simulink model "px8d1k_1g"  (R2013a) (continued)
== system px8d1k_1g == (continued)
      mask prompts: n_streams "Number simultaneous streams"; FFTSize "Size of FFT: (2^? pts)"; n_inputs "Number of Simultaneous Inputs: (2^?)"; input_bit_width "Input Bit Width"; bin_pt_in "Input binary point"; coeff_bit_width "Coefficient Bit Width"; unscramble "Unscramble output (ie, put channels in canonical order)"; async "Asynchronous operation"; add_latency "Add Latency"; mult_latency "Mult Latency"; bram_latency "BRAM Latency"; conv_latency "Convert Latency"; input_latency "Input Latency"; biplex_direct_latency "Latency between biplexes and fft_direct"; quantization "Quantization Behavior"; overflow "Overflow Behavior"; delays_bit_limit "Number bits above which to store stage's delays in BRAM (2^? bits)"; coeffs_bit_limit "Number bits above which to store stage's coefficients in BRAM (2^? bits)"; coeff_sharing "BRAM sharing in coeff storage"; coeff_decimation "Store a fraction of coeff factors where useful"; coeff_generation "Generate coeffs with multipliers where useful"; cal_bits "Number calibration locations when generating coeffs (2^?)"; n_bits_rotation "Feedback rotation vector resolution"; mult_spec "Multiplier impl. (0=core, 1=embedded, 2=behavioural) (left=1st stage, or just one value)"; max_fanout "Maximum fanout"; bitgrowth "Bitgrowth instead of shifting"; max_bits "Max bits to grow to"; hardcode_shifts "Hardcode shift schedule"; shift_schedule "Shift schedule"; dsp48_adders "DSP48 adders in butterfly"
      mask help: A real-sampled wideband FFT.
      mask init:
      | fft_wideband_real_init(gcb, ...
      |     'n_streams', n_streams, ...
      |     'FFTSize', FFTSize, ...
      |     'n_inputs', n_inputs, ...
      |     'input_bit_width', input_bit_width, ...
      |     'bin_pt_in', bin_pt_in, ...
      |     'coeff_bit_width', coeff_bit_width, ...
      |     'unscramble', unscramble, ...
      |     'async', async, ...
      |     'add_latency', add_latency, ...
      |     'mult_latency', mult_latency, ...
      |     'bram_latency', bram_latency, ...
      |     'conv_latency', conv_latency, ...
      |     'input_latency', input_latency, ...
      |     'biplex_direct_latency', biplex_direct_latency, ...
      |     'quantization', quantization, ...
      |     'overflow', overflow, ...
      |     'delays_bit_limit', delays_bit_limit, ...
      |     'coeffs_bit_limit', coeffs_bit_limit, ...
      |     'coeff_sharing', coeff_sharing, ...
      |     'coeff_decimation', coeff_decimation, ...
      |     'coeff_generation', coeff_generation, ...
      |     'cal_bits', cal_bits, ...
      |     'n_bits_rotation', n_bits_rotation, ...
      |     'max_fanout', max_fanout, ...   
      |     'mult_spec', mult_spec, ...
      |     'bitgrowth', bitgrowth, ...
      |     'max_bits', max_bits, ...
      |     'hardcode_shifts', hardcode_shifts, ...
      |     'shift_schedule', shift_schedule, ...
      |     'dsp48_adders', dsp48_adders);
      settings: AttributesFormatString=|
      | 11 stages
      | (18,18)
      | Round  (unbiased: +/- Inf)
      | Saturate
      mask Help: eval('xlWeb(''http://casper.berkeley.edu/wiki/Fft_wideband_real'')')
      mask TypeOptions quantization: Truncate | Round  (unbiased: +/- Inf) | Round  (unbiased: Even Values)
      mask TypeOptions overflow: Wrap | Saturate | Error
      mask Callback hardcode_shifts:
      | hardcode_shifts = get_param(gcb, 'hardcode_shifts');
      | set_param_state(gcb, 'shift_schedule', hardcode_shifts);
      mask Callback dsp48_adders: fft_callback_dsp48_adders(gcb);
  gain0 : Subsystem  Tag=xps:sw_reg  AncestorBlock=xps_library/software register  mask(swreg){mode=one value, io_dir=From Processor, io_delay=0, sample_period=1, names=reg, bitwidths=32, arith_types=0, bin_pts=0, sim_port=on, show_format=off}
      mask prompts: io_dir "I/O direction"; io_delay "I/O delay"; sample_period "Sample period"; names "Name:"; bitwidths "Bitwidth:"; arith_types "Type (ufix=0, fix=1, bool=2):"; bin_pts "Binary pt:"; sim_port "Provide sim input/output?"; show_format "Print format string?"
      mask init:
      | swreg_init(gcb)
      settings: AttributesFormatString=1input
      mask TypeOptions mode: one value | fields of equal size | fields of arbitrary size
      mask Callback mode: swreg_cb(gcb);
      mask TypeOptions io_dir: To Processor | From Processor
      mask Callback io_dir:
      | mask_enables = get_param(gcb, 'MaskEnables');
      | mask_names = get_param(gcb, 'MaskNames');
      | if strcmp(get_param(gcb, 'io_dir'), 'To Processor'),
      |     mask_enables{ismember(mask_names, 'sample_period')} = 'off';
      | else
      |     mask_enables{ismember(mask_names, 'sample_period')} = 'on';
      | end
      | set_param(gcb, 'MaskEnables', mask_enables);
      mask Callback bitwidths:
      | swreg_maskcheck(gcb);
      | % blk = gcb;
      | % mode = get_param(blk, 'mode');
      | % current_widths = eval(get_param(blk, 'bitwidths'));
      | % if (strcmp(mode, 'one value') || strcmp(mode, 'fields of equal size')) && (length(current_widths) > 1),
      | %     error('Only one width may be specified for this mode.');
      | % elseif strcmp(mode, 'fields of arbitrary size')
      | %     current_names = textscan(strtrim(strrep(strrep(get_param(blk, 'names'), ']', ''), '[', '')), '%s');
      | %     current_names = current_names{1};
      | %     numios = length(current_names);
      | %     if length(current_widths) ~= numios,
      | %         error('%d field names specified, but %d widths?', numios, length(current_widths));
      | %     end
      | % end
      | % total_width = 0;
      | % for ctr = 1 : length(current_widths),
      | %     total_width = total_width + current_widths(ctr);
      | % end
      | % if total_width > 32,
      | %     error('Total width must be 32 or less, set to %d.', total_width);
      | % end
      mask Callback arith_types:
      | [numios, ~, ~, ~, current_types] = swreg_maskcheck(gcb);
      | % blk = gcb;
      | % mode = get_param(blk, 'mode');
      | % current_types = eval(get_param(blk, 'arith_types'));
      | % numios = length(current_types);
      | % if (strcmp(mode, 'one value') || strcmp(mode, 'fields of equal size')) && (length(current_types) > 1),
      | %     error('Only one type may be specified for this mode.');
      | % elseif strcmp(mode, 'fields of arbitrary size')
      | %     current_names = textscan(strtrim(strrep(strrep(get_param(blk, 'names'), ']', ''), '[', '')), '%s');
      | %     current_names = current_names{1};
      | %     numios = length(current_names);
      | %     if length(current_types) ~= numios,
      | %         error('%d field names specified, but %d types?', numios, length(current_types));
      | %     end
      | % end
      | problem = 0;
      | for ctr = 1 : numios,
      |     t = current_types(ctr);
      |     if (t < 0) || (t > 2),
      |         problem = t;
      |         ctr = numios + 1;
      |     end
      | end
      | if problem ~= 0,
      |     error('Type cannot be %i, must be 0, 1 or 2.', problem);
      | end
      mask Callback bin_pts:
      | swreg_maskcheck(gcb);
      | % blk = gcb;
      | % mode = get_param(blk, 'mode');
      | % current_bins = eval(get_param(blk, 'bin_pts'));
      | % if (strcmp(mode, 'one value') || strcmp(mode, 'fields of equal size')) && (length(current_bins) > 1),
      | %     error('Only one binary pt may be specified for this mode.');
      | % elseif strcmp(mode, 'fields of arbitrary size')
      | %     current_names = textscan(strtrim(strrep(strrep(get_param(blk, 'names'), ']', ''), '[', '')), '%s');
      | %     current_names = current_names{1};
      | %     numios = length(current_names);
      | %     if length(current_bins) ~= numios,
      | %         error('%d field names specified, but %d binary pts?', numios, length(current_bins));
      | %     end
      | % end
  gain1 : Subsystem  Tag=xps:sw_reg  AncestorBlock=xps_library/software register  mask(swreg){mode=one value, io_dir=From Processor, io_delay=0, sample_period=1, names=reg, bitwidths=32, arith_types=0, bin_pts=0, sim_port=on, show_format=off}
      mask prompts: io_dir "I/O direction"; io_delay "I/O delay"; sample_period "Sample period"; names "Name:"; bitwidths "Bitwidth:"; arith_types "Type (ufix=0, fix=1, bool=2):"; bin_pts "Binary pt:"; sim_port "Provide sim input/output?"; show_format "Print format string?"
      mask init:
      | swreg_init(gcb)
      settings: AttributesFormatString=1input
      mask TypeOptions mode: one value | fields of equal size | fields of arbitrary size
      mask Callback mode: swreg_cb(gcb);
      mask TypeOptions io_dir: To Processor | From Processor
      mask Callback io_dir:
      | mask_enables = get_param(gcb, 'MaskEnables');
      | mask_names = get_param(gcb, 'MaskNames');
      | if strcmp(get_param(gcb, 'io_dir'), 'To Processor'),
      |     mask_enables{ismember(mask_names, 'sample_period')} = 'off';
      | else
      |     mask_enables{ismember(mask_names, 'sample_period')} = 'on';
      | end
      | set_param(gcb, 'MaskEnables', mask_enables);
      mask Callback bitwidths:
      | swreg_maskcheck(gcb);
      | % blk = gcb;
      | % mode = get_param(blk, 'mode');
      | % current_widths = eval(get_param(blk, 'bitwidths'));
      | % if (strcmp(mode, 'one value') || strcmp(mode, 'fields of equal size')) && (length(current_widths) > 1),
      | %     error('Only one width may be specified for this mode.');
      | % elseif strcmp(mode, 'fields of arbitrary size')
      | %     current_names = textscan(strtrim(strrep(strrep(get_param(blk, 'names'), ']', ''), '[', '')), '%s');
      | %     current_names = current_names{1};
      | %     numios = length(current_names);
      | %     if length(current_widths) ~= numios,
      | %         error('%d field names specified, but %d widths?', numios, length(current_widths));
      | %     end
      | % end
      | % total_width = 0;
      | % for ctr = 1 : length(current_widths),
      | %     total_width = total_width + current_widths(ctr);
      | % end
      | % if total_width > 32,
      | %     error('Total width must be 32 or less, set to %d.', total_width);
      | % end
      mask Callback arith_types:
      | [numios, ~, ~, ~, current_types] = swreg_maskcheck(gcb);
      | % blk = gcb;
      | % mode = get_param(blk, 'mode');
      | % current_types = eval(get_param(blk, 'arith_types'));
      | % numios = length(current_types);
      | % if (strcmp(mode, 'one value') || strcmp(mode, 'fields of equal size')) && (length(current_types) > 1),
      | %     error('Only one type may be specified for this mode.');
      | % elseif strcmp(mode, 'fields of arbitrary size')
      | %     current_names = textscan(strtrim(strrep(strrep(get_param(blk, 'names'), ']', ''), '[', '')), '%s');
      | %     current_names = current_names{1};
      | %     numios = length(current_names);
      | %     if length(current_types) ~= numios,
      | %         error('%d field names specified, but %d types?', numios, length(current_types));
      | %     end
      | % end
      | problem = 0;
      | for ctr = 1 : numios,
      |     t = current_types(ctr);
      |     if (t < 0) || (t > 2),
      |         problem = t;
      |         ctr = numios + 1;
      |     end
      | end
      | if problem ~= 0,
      |     error('Type cannot be %i, must be 0, 1 or 2.', problem);
      | end
      mask Callback bin_pts:
      | swreg_maskcheck(gcb);
      | % blk = gcb;
      | % mode = get_param(blk, 'mode');
      | % current_bins = eval(get_param(blk, 'bin_pts'));
      | % if (strcmp(mode, 'one value') || strcmp(mode, 'fields of equal size')) && (length(current_bins) > 1),
      | %     error('Only one binary pt may be specified for this mode.');
      | % elseif strcmp(mode, 'fields of arbitrary size')
      | %     current_names = textscan(strtrim(strrep(strrep(get_param(blk, 'names'), ']', ''), '[', '')), '%s');
      | %     current_names = current_names{1};
      | %     numios = length(current_names);
      | %     if length(current_bins) ~= numios,
      | %         error('%d field names specified, but %d binary pts?', numios, length(current_bins));
      | %     end
      | % end
  one_GbE : xps_library/one_GbE  Tag=xps:onegbe  local_en=on  dis_cpu_rx=off  dis_cpu_tx=off  cpu_promiscuous=off  local_mac=hex2dec('123456789abc')  local_ip=hex2dec('c0a8290a')  local_port=4321  local_gateway=1
  pfb_fir_real : Subsystem  Tag=casper:pfb_fir_real  AncestorBlock=casper_library_pfbs/pfb_fir_real  mask(pfb_fir_real){PFBSize=11, TotalTaps=4, WindowType=hamming, n_inputs=3, MakeBiplex=on, BitWidthIn=8, BitWidthOut=18, CoeffBitWidth=18, CoeffDistMem=off, add_latency=3, mult_latency=4, bram_latency=4, fan_latency=2, conv_latency=2, quantization=Round  (unbiased: Even Values), fwidth=1, mult_spec=2, adder_folding=off, adder_imp=Behavioral, coeffs_share=on}
      mask prompts: PFBSize "Size of PFB: (2^? pnts)"; TotalTaps "Total Number of Taps:"; WindowType "Windowing Function:"; n_inputs "Number of Simultaneous Inputs: (2^?)"; MakeBiplex "Make Biplex?"; BitWidthIn "Input Bitwidth:"; BitWidthOut "Output Bitwidth:"; CoeffBitWidth "Coefficient Bitwidth:"; CoeffDistMem "Use Distributed Memory for Coeffs"; add_latency "Add Latency"; mult_latency "Mult Latency"; bram_latency "BRAM Latency"; fan_latency "Fanout latency"; conv_latency "Convert latency"; quantization "Quantization Behavior"; fwidth "Bin Width Scaling (normal=1)"; mult_spec "Multiplier specification (0=core, 1=embedded, 2=behavioural) (left=1st tap, or just one value)"; adder_folding "Fold adders into DSPs"; adder_imp "Adder implementation"; coeffs_share "Share coefficients between polarisations (use less BRAM)?"
      mask help:
      | Fold adders into DSPs: Causes adders to be absorbed into DSP blocks (supported in Virtex5)
      | Adder implementation: Cores using Fabric or DSP48 or behavioral HDL
      mask init:
      | pfb_fir_real_init(gcb, ...
      |     'PFBSize', PFBSize, ...
      |     'TotalTaps', TotalTaps, ...
      |     'WindowType', WindowType, ...
      |     'n_inputs', n_inputs, ...
      |     'MakeBiplex', MakeBiplex, ...
      |     'BitWidthIn', BitWidthIn, ...
      |     'BitWidthOut', BitWidthOut, ...
      |     'CoeffBitWidth', CoeffBitWidth, ...
      |     'CoeffDistMem', CoeffDistMem, ...
      |     'add_latency', add_latency, ...
      |     'mult_latency', mult_latency, ...
      |     'bram_latency', bram_latency, ...
      |     'fan_latency', fan_latency, ...
      |     'conv_latency', conv_latency, ...
      |     'quantization', quantization, ...
      |     'fwidth', fwidth, ...
      |     'mult_spec', mult_spec, ...
      |     'adder_folding', adder_folding, ...
      |     'adder_imp', adder_imp, ...
      |     'coeffs_share', coeffs_share);
      settings: AttributesFormatString=taps=4, add_latency=3
      mask Help: eval('xlWeb(''http://casper.berkeley.edu/wiki/Pfb_fir_real'')')
      mask Callback TotalTaps:
      | %num_taps = eval(get_param(gcb, 'TotalTaps'));
      | %old_vec = eval(get_param(gcb, 'mult_spec'));=-0l
      | 
      | %vec = 2.*ones(1,num_taps);
      | %old_size = length(old_vec);
      | 
      | %if(old_size <= num_taps),
      | %    new_size = old_size;
      | %else,
      | %    new_size = num_taps;
      | %end
      | 
      | %for n = 1:new_size, vec(n) = old_vec(n); end
      | %set_param(gcb, 'mult_spec', mat2str(vec));
      mask TypeOptions WindowType: bartlett | barthannwin | blackman | blackmanharris | bohamwin | chebwin | flattopwin | gausswin | hamming | hann | kaiser | nuttallwin | parzenwin | rectwin | tukeywin | triang | userwindow
      mask TypeOptions quantization: Truncate | Round  (unbiased: +/- Inf) | Round  (unbiased: Even Values)
      mask TypeOptions adder_imp: Behavioral | Fabric | DSP48
  pipeline5 : xbsIndex_r4/Delay  rst=off  infoeditControl=Selection of Reset will increase slice count due to use of real FFs and instead of SRLs  en=off  latency=1  dbl_ovrd=off  reg_retiming=on  block_type=delay
      settings: xl_area=[0, 0, 0, 0, 0, 0, 0]  block_version=11.4
  pkt_64_to_8 : Subsystem  mask{pktlen=2^8, idlecycles=64}
      mask prompts: pktlen "Packet length in INPUT word (64bit):"; idlecycles "Idle cycles between packet:"
  power : Subsystem  mask{bitwidth=18, add_latency=2, mult_latency=3, mode=dsp48e, n_inputs=8}
      mask prompts: bitwidth "Bit Width"; add_latency "Adder Latency"; mult_latency "Mult Latency"; n_inputs "Number of Inputs"
      mask init:
      | config.source = str2func('spect_power');
      | config.depend = {'spect_power.m'};
      | config.toplevel = gcb;
      | xBlock(config, {bitwidth, add_latency, mult_latency, mode, n_inputs});
      mask TypeOptions mode: behavioral | dsp48e
  ppsarm : Subsystem
  prescale : Subsystem  mask{mult_latency=3}
      mask prompts: mult_latency "Mult Latency"
  reset : Subsystem  Tag=xps:sw_reg  AncestorBlock=xps_library/software register  mask(swreg){mode=one value, io_dir=From Processor, io_delay=0, sample_period=1, names=reg, bitwidths=32, arith_types=2, bin_pts=0, sim_port=on, show_format=off}
      mask prompts: io_dir "I/O direction"; io_delay "I/O delay"; sample_period "Sample period"; names "Name:"; bitwidths "Bitwidth:"; arith_types "Type (ufix=0, fix=1, bool=2):"; bin_pts "Binary pt:"; sim_port "Provide sim input/output?"; show_format "Print format string?"
      mask init:
      | swreg_init(gcb)
      settings: AttributesFormatString=1input
      mask TypeOptions mode: one value | fields of equal size | fields of arbitrary size
      mask Callback mode: swreg_cb(gcb);
      mask TypeOptions io_dir: To Processor | From Processor
      mask Callback io_dir:
      | mask_enables = get_param(gcb, 'MaskEnables');
      | mask_names = get_param(gcb, 'MaskNames');
      | if strcmp(get_param(gcb, 'io_dir'), 'To Processor'),
      |     mask_enables{ismember(mask_names, 'sample_period')} = 'off';
      | else
      |     mask_enables{ismember(mask_names, 'sample_period')} = 'on';
      | end
      | set_param(gcb, 'MaskEnables', mask_enables);
      mask Callback bitwidths:
      | swreg_maskcheck(gcb);
      | % blk = gcb;
      | % mode = get_param(blk, 'mode');
      | % current_widths = eval(get_param(blk, 'bitwidths'));
      | % if (strcmp(mode, 'one value') || strcmp(mode, 'fields of equal size')) && (length(current_widths) > 1),
      | %     error('Only one width may be specified for this mode.');
      | % elseif strcmp(mode, 'fields of arbitrary size')
      | %     current_names = textscan(strtrim(strrep(strrep(get_param(blk, 'names'), ']', ''), '[', '')), '%s');
      | %     current_names = current_names{1};
      | %     numios = length(current_names);
      | %     if length(current_widths) ~= numios,
      | %         error('%d field names specified, but %d widths?', numios, length(current_widths));
      | %     end
      | % end
      | % total_width = 0;
      | % for ctr = 1 : length(current_widths),
      | %     total_width = total_width + current_widths(ctr);
      | % end
      | % if total_width > 32,
      | %     error('Total width must be 32 or less, set to %d.', total_width);
      | % end
      mask Callback arith_types:
      | [numios, ~, ~, ~, current_types] = swreg_maskcheck(gcb);
      | % blk = gcb;
      | % mode = get_param(blk, 'mode');
      | % current_types = eval(get_param(blk, 'arith_types'));
      | % numios = length(current_types);
      | % if (strcmp(mode, 'one value') || strcmp(mode, 'fields of equal size')) && (length(current_types) > 1),
      | %     error('Only one type may be specified for this mode.');
      | % elseif strcmp(mode, 'fields of arbitrary size')
      | %     current_names = textscan(strtrim(strrep(strrep(get_param(blk, 'names'), ']', ''), '[', '')), '%s');
      | %     current_names = current_names{1};
      | %     numios = length(current_names);
      | %     if length(current_types) ~= numios,
      | %         error('%d field names specified, but %d types?', numios, length(current_types));
      | %     end
      | % end
      | problem = 0;
      | for ctr = 1 : numios,
      |     t = current_types(ctr);
      |     if (t < 0) || (t > 2),
      |         problem = t;
      |         ctr = numios + 1;
      |     end
      | end
      | if problem ~= 0,
      |     error('Type cannot be %i, must be 0, 1 or 2.', problem);
      | end
      mask Callback bin_pts:
      | swreg_maskcheck(gcb);
      | % blk = gcb;
      | % mode = get_param(blk, 'mode');
      | % current_bins = eval(get_param(blk, 'bin_pts'));
      | % if (strcmp(mode, 'one value') || strcmp(mode, 'fields of equal size')) && (length(current_bins) > 1),
      | %     error('Only one binary pt may be specified for this mode.');
      | % elseif strcmp(mode, 'fields of arbitrary size')
      | %     current_names = textscan(strtrim(strrep(strrep(get_param(blk, 'names'), ']', ''), '[', '')), '%s');
      | %     current_names = current_names{1};
      | %     numios = length(current_names);
      | %     if length(current_bins) ~= numios,
      | %         error('%d field names specified, but %d binary pts?', numios, length(current_bins));
      | %     end
      | % end
  scope0 : Subsystem  Tag=casper:snapshot  AncestorBlock=casper_library_scopes/snapshot  mask(snapshot){storage=bram, dram_dimm=1, dram_clock=200, nsamples=8, data_width=128, offset=off, circap=off, value=off, use_dsp48=on}
      mask prompts: storage "Storage medium"; dram_dimm "DRAM dimm"; dram_clock "DRAM clock rate"; nsamples "Number of Samples (2^?)"; data_width "Data width"; offset "Start delay support"; circap "Circular capture support"; value "Extra value capture support"; use_dsp48 "Use DSP48s to implement counters"
      mask help:
      | Standard snap block, but with the following optional extras;
      |  1) Optionally delay the fabric trigger by a user-specified number of valids.
      |  2) Capture continuously after a trigger until a stop pulse is received. Data is captured into BRAM continuously, with old data being overwritten until a stop command is received. Then stop capturing when memory is filled. This enables you to get some data before and some data after the stop pulse. Use the 'tr_en_cnt' register to determine where you are in your vector. 'Addr' register's MSb now indicates 'busy capturing'.
      |  3) Optionally capture a value on an input port to a register at the same time as the first data sample.
      |  4) Implement counters using DSP48Es to save resources.
      mask init: same as px8d1k_1g/adc_scope0
      mask TypeOptions storage: bram | dram
      mask Callback storage: snapshot_callback();
      mask TypeOptions dram_dimm: 1 | 2 | 3 | 4
      mask TypeOptions dram_clock: 200 | 250 | 266 | 300 | 333
      mask TypeOptions data_width: 8 | 16 | 32 | 64 | 128
  scope1 : Subsystem  Tag=casper:snapshot  AncestorBlock=casper_library_scopes/snapshot  mask(snapshot){storage=bram, dram_dimm=1, dram_clock=200, nsamples=8, data_width=128, offset=off, circap=off, value=off, use_dsp48=on}
      mask prompts: storage "Storage medium"; dram_dimm "DRAM dimm"; dram_clock "DRAM clock rate"; nsamples "Number of Samples (2^?)"; data_width "Data width"; offset "Start delay support"; circap "Circular capture support"; value "Extra value capture support"; use_dsp48 "Use DSP48s to implement counters"
      mask help:
      | Standard snap block, but with the following optional extras;
      |  1) Optionally delay the fabric trigger by a user-specified number of valids.
      |  2) Capture continuously after a trigger until a stop pulse is received. Data is captured into BRAM continuously, with old data being overwritten until a stop command is received. Then stop capturing when memory is filled. This enables you to get some data before and some data after the stop pulse. Use the 'tr_en_cnt' register to determine where you are in your vector. 'Addr' register's MSb now indicates 'busy capturing'.
      |  3) Optionally capture a value on an input port to a register at the same time as the first data sample.
      |  4) Implement counters using DSP48Es to save resources.
      mask init: same as px8d1k_1g/adc_scope0
      mask TypeOptions storage: bram | dram
      mask Callback storage: snapshot_callback();
      mask TypeOptions dram_dimm: 1 | 2 | 3 | 4
      mask TypeOptions dram_clock: 200 | 250 | 266 | 300 | 333
      mask TypeOptions data_width: 8 | 16 | 32 | 64 | 128
  sim_acclen : Constant  Value=2
  sim_bitsel : Constant
  sim_destip : Constant  Value=10*2^24+123
  sim_destport : Constant  Value=33333
  sim_fftshift : Constant  Value=2^32-1
  sim_gain0 : Constant  Value=2^12
  sim_gain1 : Constant  Value=2^12
  sim_usetvg : Constant
  status : Subsystem  Tag=xps:sw_reg  AncestorBlock=xps_library/software register  mask(swreg){mode=fields of arbitrary size, io_dir=To Processor, io_delay=0, sample_period=1, names=[unused tx_overrun pkt_overrun fft_overflow], bitwidths=[26 1 1 4], arith_types=[0 2 2 0], bin_pts=0, sim_port=off, show_format=off}
      mask prompts: io_dir "I/O direction"; io_delay "I/O delay"; sample_period "Sample period"; names "Names [msb ... lsb]:"; bitwidths "Bitwidths [msb ... lsb]:"; arith_types "Types (ufix=0, fix=1, bool=2) [msb ... lsb]:"; bin_pts "Binary pts [msb ... lsb]:"; sim_port "Provide sim input/output?"; show_format "Print format string?"
      mask init:
      | swreg_init(gcb)
      settings: AttributesFormatString=4 outputs
      mask TypeOptions mode: one value | fields of equal size | fields of arbitrary size
      mask Callback mode: swreg_cb(gcb);
      mask TypeOptions io_dir: To Processor | From Processor
      mask Callback io_dir:
      | mask_enables = get_param(gcb, 'MaskEnables');
      | mask_names = get_param(gcb, 'MaskNames');
      | if strcmp(get_param(gcb, 'io_dir'), 'To Processor'),
      |     mask_enables{ismember(mask_names, 'sample_period')} = 'off';
      | else
      |     mask_enables{ismember(mask_names, 'sample_period')} = 'on';
      | end
      | set_param(gcb, 'MaskEnables', mask_enables);
      mask Callback bitwidths:
      | swreg_maskcheck(gcb);
      | % blk = gcb;
      | % mode = get_param(blk, 'mode');
      | % current_widths = eval(get_param(blk, 'bitwidths'));
      | % if (strcmp(mode, 'one value') || strcmp(mode, 'fields of equal size')) && (length(current_widths) > 1),
      | %     error('Only one width may be specified for this mode.');
      | % elseif strcmp(mode, 'fields of arbitrary size')
      | %     current_names = textscan(strtrim(strrep(strrep(get_param(blk, 'names'), ']', ''), '[', '')), '%s');
      | %     current_names = current_names{1};
      | %     numios = length(current_names);
      | %     if length(current_widths) ~= numios,
      | %         error('%d field names specified, but %d widths?', numios, length(current_widths));
      | %     end
      | % end
      | % total_width = 0;
      | % for ctr = 1 : length(current_widths),
      | %     total_width = total_width + current_widths(ctr);
      | % end
      | % if total_width > 32,
      | %     error('Total width must be 32 or less, set to %d.', total_width);
      | % end
      mask Callback arith_types:
      | [numios, ~, ~, ~, current_types] = swreg_maskcheck(gcb);
      | % blk = gcb;
      | % mode = get_param(blk, 'mode');
      | % current_types = eval(get_param(blk, 'arith_types'));
      | % numios = length(current_types);
      | % if (strcmp(mode, 'one value') || strcmp(mode, 'fields of equal size')) && (length(current_types) > 1),
      | %     error('Only one type may be specified for this mode.');
      | % elseif strcmp(mode, 'fields of arbitrary size')
      | %     current_names = textscan(strtrim(strrep(strrep(get_param(blk, 'names'), ']', ''), '[', '')), '%s');
      | %     current_names = current_names{1};
      | %     numios = length(current_names);
      | %     if length(current_types) ~= numios,
      | %         error('%d field names specified, but %d types?', numios, length(current_types));
      | %     end
      | % end
      | problem = 0;
      | for ctr = 1 : numios,
      |     t = current_types(ctr);
      |     if (t < 0) || (t > 2),
      |         problem = t;
      |         ctr = numios + 1;
      |     end
      | end
      | if problem ~= 0,
      |     error('Type cannot be %i, must be 0, 1 or 2.', problem);
      | end
      mask Callback bin_pts:
      | swreg_maskcheck(gcb);
      | % blk = gcb;
      | % mode = get_param(blk, 'mode');
      | % current_bins = eval(get_param(blk, 'bin_pts'));
      | % if (strcmp(mode, 'one value') || strcmp(mode, 'fields of equal size')) && (length(current_bins) > 1),
      | %     error('Only one binary pt may be specified for this mode.');
      | % elseif strcmp(mode, 'fields of arbitrary size')
      | %     current_names = textscan(strtrim(strrep(strrep(get_param(blk, 'names'), ']', ''), '[', '')), '%s');
      | %     current_names = current_names{1};
      | %     numios = length(current_names);
      | %     if length(current_bins) ~= numios,
      | %         error('%d field names specified, but %d binary pts?', numios, length(current_bins));
      | %     end
      | % end
  step_arm : Step  After=7  SampleTime=0
  step_reset : Step  SampleTime=0
  use_tvg : Subsystem  Tag=xps:sw_reg  AncestorBlock=xps_library/software register  mask(swreg){mode=one value, io_dir=From Processor, io_delay=0, sample_period=1, names=reg, bitwidths=32, arith_types=2, bin_pts=0, sim_port=on, show_format=off}
      mask prompts: io_dir "I/O direction"; io_delay "I/O delay"; sample_period "Sample period"; names "Name:"; bitwidths "Bitwidth:"; arith_types "Type (ufix=0, fix=1, bool=2):"; bin_pts "Binary pt:"; sim_port "Provide sim input/output?"; show_format "Print format string?"
      mask init:
      | swreg_init(gcb)
      settings: AttributesFormatString=1input
      mask TypeOptions mode: one value | fields of equal size | fields of arbitrary size
      mask Callback mode: swreg_cb(gcb);
      mask TypeOptions io_dir: To Processor | From Processor
      mask Callback io_dir:
      | mask_enables = get_param(gcb, 'MaskEnables');
      | mask_names = get_param(gcb, 'MaskNames');
      | if strcmp(get_param(gcb, 'io_dir'), 'To Processor'),
      |     mask_enables{ismember(mask_names, 'sample_period')} = 'off';
      | else
      |     mask_enables{ismember(mask_names, 'sample_period')} = 'on';
      | end
      | set_param(gcb, 'MaskEnables', mask_enables);
      mask Callback bitwidths:
      | swreg_maskcheck(gcb);
      | % blk = gcb;
      | % mode = get_param(blk, 'mode');
      | % current_widths = eval(get_param(blk, 'bitwidths'));
      | % if (strcmp(mode, 'one value') || strcmp(mode, 'fields of equal size')) && (length(current_widths) > 1),
      | %     error('Only one width may be specified for this mode.');
      | % elseif strcmp(mode, 'fields of arbitrary size')
      | %     current_names = textscan(strtrim(strrep(strrep(get_param(blk, 'names'), ']', ''), '[', '')), '%s');
      | %     current_names = current_names{1};
      | %     numios = length(current_names);
      | %     if length(current_widths) ~= numios,
      | %         error('%d field names specified, but %d widths?', numios, length(current_widths));
      | %     end
      | % end
      | % total_width = 0;
      | % for ctr = 1 : length(current_widths),
      | %     total_width = total_width + current_widths(ctr);
      | % end
      | % if total_width > 32,
      | %     error('Total width must be 32 or less, set to %d.', total_width);
      | % end
      mask Callback arith_types:
      | [numios, ~, ~, ~, current_types] = swreg_maskcheck(gcb);
      | % blk = gcb;
      | % mode = get_param(blk, 'mode');
      | % current_types = eval(get_param(blk, 'arith_types'));
      | % numios = length(current_types);
      | % if (strcmp(mode, 'one value') || strcmp(mode, 'fields of equal size')) && (length(current_types) > 1),
      | %     error('Only one type may be specified for this mode.');
      | % elseif strcmp(mode, 'fields of arbitrary size')
      | %     current_names = textscan(strtrim(strrep(strrep(get_param(blk, 'names'), ']', ''), '[', '')), '%s');
      | %     current_names = current_names{1};
      | %     numios = length(current_names);
      | %     if length(current_types) ~= numios,
      | %         error('%d field names specified, but %d types?', numios, length(current_types));
      | %     end
      | % end
      | problem = 0;
      | for ctr = 1 : numios,
      |     t = current_types(ctr);
      |     if (t < 0) || (t > 2),
      |         problem = t;
      |         ctr = numios + 1;
      |     end
      | end
      | if problem ~= 0,
      |     error('Type cannot be %i, must be 0, 1 or 2.', problem);
      | end
      mask Callback bin_pts:
      | swreg_maskcheck(gcb);
      | % blk = gcb;
      | % mode = get_param(blk, 'mode');
      | % current_bins = eval(get_param(blk, 'bin_pts'));
      | % if (strcmp(mode, 'one value') || strcmp(mode, 'fields of equal size')) && (length(current_bins) > 1),
      | %     error('Only one binary pt may be specified for this mode.');
      | % elseif strcmp(mode, 'fields of arbitrary size')
      | %     current_names = textscan(strtrim(strrep(strrep(get_param(blk, 'names'), ']', ''), '[', '')), '%s');
      | %     current_names = current_names{1};
      | %     numios = length(current_names);
      | %     if length(current_bins) ~= numios,
      | %         error('%d field names specified, but %d binary pts?', numios, length(current_bins));
      | %     end
      | % end
  vacc : Subsystem  AncestorBlock=gavrt_library/vacc  mask(vacc){veclen=8, ninputs=8, max_accum=20, arith_type=0, in_bit_width=36, in_bin_pt=34, out_bit_width=32, out_bin_pt=23, register_din=1, add_latency=3, bram_latency=3, mux_latency=2}
      mask prompts: veclen "Vector Length (2^?)"; ninputs "Number of Simultaneous Vectors"; max_accum "Maximum # Accumulations (2^?)"; arith_type "Arithmetic Type (0=Unsigned, 1=Signed)"; in_bit_width "Input Bit Width"; in_bin_pt "Input Binary Point"; out_bit_width "Output Bit Width"; out_bin_pt "Output Binary Point"; register_din "Register Input Data  (1 = Enable Register Retiming on input delay)"; add_latency "Add Latency"; bram_latency "BRAM Latency"; mux_latency "Mux Latency"
      mask help:
      | Sync is expected the clock before the first data to be accumulated.
      | Use the Cram block to feed din for multiple simultaneous inputs.
      | acc_len is the number of vectors to sum.
      | valid is high while a valid accumulation is being clocked out on dout.
      | If only one sync pulse is provided, valid accumulations will repeatedly appear on dout as they become available.
      | Undesired behavior may occur if acc_len is changed without a new sync pulse.
      | Note: The output is an unsigned concatenation of the output streams. Thus, even with only one stream, the output must be recast before using.
      | Register Input Data controls the "Enable Register Retiming" parameter of the delays on 'din'.
      mask init:
      | vacc_init(gcb, ...
      |     'veclen', veclen, ...
      |     'ninputs', ninputs, ...
      |     'max_accum', max_accum, ...
      |     'arith_type', arith_type, ...
      |     'in_bit_width', in_bit_width, ...
      |     'in_bin_pt', in_bin_pt, ...
      |     'out_bit_width', out_bit_width, ...
      |     'out_bin_pt', out_bin_pt, ...
      |     'register_din', register_din, ...
      |     'add_latency', add_latency, ...
      |     'bram_latency', bram_latency, ...
      |     'mux_latency', mux_latency);
      settings: AttributesFormatString=|
      | vector length=256, inputs=8
      | max accumulations=2^20
  wires:
  step_reset/1 -> reset/1(sim_1)
  reset/1(in_reg) -> Delay19/1
  edge_detect/1 -> [sys_reset]
  [adc_in] -> bus_expand/1(bus_in)
  Sine Wave1/1 -> asiaa_adc5g/1(sim_a)
  Pulse Generator1/1 -> asiaa_adc5g/3(sim_sync)
  asiaa_adc5g/1(a0) -> Delay2/1
  asiaa_adc5g/2(a1) -> Delay3/1
  asiaa_adc5g/3(a2) -> Delay4/1
  asiaa_adc5g/4(a3) -> Delay5/1
  asiaa_adc5g/5(a4) -> Delay6/1
  asiaa_adc5g/6(a5) -> Delay7/1
  asiaa_adc5g/7(a6) -> Delay8/1
  asiaa_adc5g/8(a7) -> Delay9/1
  asiaa_adc5g/14(c5) -> Delay15/1
  asiaa_adc5g/16(c7) -> Delay17/1
  asiaa_adc5g/13(c4) -> Delay14/1
  asiaa_adc5g/12(c3) -> Delay13/1
  asiaa_adc5g/15(c6) -> Delay16/1
  asiaa_adc5g/11(c2) -> Delay12/1
  asiaa_adc5g/10(c1) -> Delay11/1
  asiaa_adc5g/9(c0) -> Delay10/1
  conv_obbus/11(q10) -> bus_create1/11(in11)
  conv_obbus/9(q8) -> bus_create1/9(in9)
  conv_obbus/10(q9) -> bus_create1/10(in10)
  conv_obbus/14(q13) -> bus_create1/14(in14)
  conv_obbus/15(q14) -> bus_create1/15(in15)
  conv_obbus/16(q15) -> bus_create1/16(in16)
  conv_obbus/13(q12) -> bus_create1/13(in13)
  conv_obbus/12(q11) -> bus_create1/12(in12)
  conv_obbus/1(q0) -> bus_create1/1(in1)
  conv_obbus/2(q1) -> bus_create1/2(in2)
  conv_obbus/3(q2) -> bus_create1/3(in3)
  conv_obbus/4(q3) -> bus_create1/4(in4)
  conv_obbus/5(q4) -> bus_create1/5(in5)
  conv_obbus/6(q5) -> bus_create1/6(in6)
  conv_obbus/7(q6) -> bus_create1/7(in7)
  conv_obbus/8(q7) -> bus_create1/8(in8)
  bus_create1/1(bus_out) -> Delay29/1
  [reset] -> pfb_fir_real/1(sync)
  bus_expand/2(out15) -> pfb_fir_real/3(pol1_in2)
  bus_expand/1(msb_out16) -> pfb_fir_real/2(pol1_in1)
  bus_expand/10(out7) -> pfb_fir_real/11(pol2_in2)
  bus_expand/11(out6) -> pfb_fir_real/12(pol2_in3)
  bus_expand/12(out5) -> pfb_fir_real/13(pol2_in4)
  bus_expand/13(out4) -> pfb_fir_real/14(pol2_in5)
  bus_expand/14(out3) -> pfb_fir_real/15(pol2_in6)
  bus_expand/15(out2) -> pfb_fir_real/16(pol2_in7)
  bus_expand/16(lsb_out1) -> pfb_fir_real/17(pol2_in8)
  bus_expand/9(out8) -> pfb_fir_real/10(pol2_in1)
  bus_expand/4(out13) -> pfb_fir_real/5(pol1_in4)
  bus_expand/8(out9) -> pfb_fir_real/9(pol1_in8)
  bus_expand/3(out14) -> pfb_fir_real/4(pol1_in3)
  bus_expand/6(out11) -> pfb_fir_real/7(pol1_in6)
  bus_expand/5(out12) -> pfb_fir_real/6(pol1_in5)
  bus_expand/7(out10) -> pfb_fir_real/8(pol1_in7)
  pfb_fir_real/1(sync_out) -> fft_wideband_real/1(sync)
  [fft_shift] -> fft_wideband_real/2(shift)
  pfb_fir_real/17(pol2_out8) -> fft_wideband_real/18(in17)
  pfb_fir_real/16(pol2_out7) -> fft_wideband_real/17(in16)
  pfb_fir_real/15(pol2_out6) -> fft_wideband_real/16(in15)
  pfb_fir_real/14(pol2_out5) -> fft_wideband_real/15(in14)
  pfb_fir_real/13(pol2_out4) -> fft_wideband_real/14(in13)
  pfb_fir_real/12(pol2_out3) -> fft_wideband_real/13(in12)
  pfb_fir_real/11(pol2_out2) -> fft_wideband_real/12(in11)
  pfb_fir_real/10(pol2_out1) -> fft_wideband_real/11(in10)
  pfb_fir_real/2(pol1_out1) -> fft_wideband_real/3(in00)
  pfb_fir_real/3(pol1_out2) -> fft_wideband_real/4(in01)
  pfb_fir_real/4(pol1_out3) -> fft_wideband_real/5(in02)
  pfb_fir_real/5(pol1_out4) -> fft_wideband_real/6(in03)
  pfb_fir_real/6(pol1_out5) -> fft_wideband_real/7(in04)
  pfb_fir_real/8(pol1_out7) -> fft_wideband_real/9(in06)
  pfb_fir_real/7(pol1_out6) -> fft_wideband_real/8(in05)
  pfb_fir_real/9(pol1_out8) -> fft_wideband_real/10(in07)
  fft_wideband_real/1(sync_out) -> prescale/3(sync_in)
  fft_wideband_real/2(out00) -> prescale/4(din00)
  fft_wideband_real/3(out01) -> prescale/5(din01)
  fft_wideband_real/4(out02) -> prescale/6(din02)
  fft_wideband_real/5(out03) -> prescale/7(din03)
  fft_wideband_real/6(out10) -> prescale/8(din10)
  fft_wideband_real/7(out11) -> prescale/9(din11)
  fft_wideband_real/8(out12) -> prescale/10(din12)
  fft_wideband_real/9(out13) -> prescale/11(din13)
  prescale/5(dout03) -> power/5(din_3)
  prescale/4(dout02) -> power/4(din_2)
  prescale/6(dout10) -> power/6(din_4)
  prescale/8(dout12) -> power/8(din_6)
  prescale/9(dout13) -> power/9(din_7)
  prescale/7(dout11) -> power/7(din_5)
  prescale/1(sync_out) -> power/1(sync_in)
  prescale/3(dout01) -> power/3(din_1)
  prescale/2(dout00) -> power/2(din_0)
  gain0/1(in_reg) -> Delay23/1
  gain1/1(in_reg) -> Delay24/1
  sim_gain0/1 -> gain0/1(sim_1)
  sim_gain1/1 -> gain1/1(sim_1)
  [gain0] -> prescale/1(coeff0)
  [gain1] -> prescale/2(coeff1)
  power/1(sync_out) -> Gateway Out29/1, vacc/1(sync), Delay30/1
  bus_create2/1(bus_out) -> bus_expand3/1(bus_in), vacc/2(din)
  power/2(dout_0) -> bus_create2/1(in1)
  power/3(dout_1) -> bus_create2/2(in2)
  power/4(dout_2) -> bus_create2/3(in3)
  power/5(dout_3) -> bus_create2/4(in4)
  power/6(dout_4) -> bus_create2/5(in5)
  power/7(dout_5) -> bus_create2/6(in6)
  power/8(dout_6) -> bus_create2/7(in7)
  power/9(dout_7) -> bus_create2/8(in8)
  acc_len/1(in_reg) -> Delay25/1
  sim_acclen/1 -> acc_len/1(sim_1)
  [acc_len] -> vacc/3(acc_len)
  vacc/1(valid) -> [vacc_valid]
  vacc/2(dout) -> bitselect/2(data_in), Slice1/1, Slice6/1
  [vacc_valid] -> Delay/1, edge_detect1/1(in)
  edge_detect1/1(out) -> Gateway Out10/1, scope0/3(trig)
  Slice6/1 -> Delay32/1
  Delay/1 -> Gateway Out9/1, scope0/2(we)
  [vacc_valid] -> Delay1/1, edge_detect2/1(in)
  Slice1/1 -> Delay33/1
  Delay1/1 -> scope1/2(we)
  edge_detect2/1(out) -> scope1/3(trig)
  bit_select/1(in_reg) -> Delay22/1
  sim_bitsel/1 -> bit_select/1(sim_1)
  [bitsel] -> bitselect/1(bitselect)
  [vacc_valid] -> pipeline5/1
  pipeline5/1 -> pkt_64_to_8/3(din_valid)
  pkt_64_to_8/1(dout) -> Gateway Out3/1, one_GbE/3
  pkt_64_to_8/2(dout_valid) -> Gateway Out1/1, one_GbE/4
  pkt_64_to_8/3(eof) -> Gateway Out2/1, one_GbE/7
  pkt_64_to_8/4(overflow) -> [pkt_overrun]
  bitselect/1(data_out) -> pkt_64_to_8/2(din)
  [reset1] -> pkt_64_to_8/1(reset)
  dest_ip/1(in_reg) -> Delay18/1
  sim_destip/1 -> dest_ip/1(sim_1)
  dest_port/1(in_reg) -> Delay20/1
  sim_destport/1 -> dest_port/1(sim_1)
  fft_wideband_real/10(of) -> [fft_overflow]
  [fft_overflow] -> Delay26/1
  [pkt_overrun] -> Delay27/1
  [gbe_overrun] -> Delay28/1
  Constant2/1 -> status/1(out_unused)
  one_GbE/2 -> [gbe_overrun]
  [dest_ip] -> one_GbE/5
  [dest_port] -> one_GbE/6
  Constant3/1 -> one_GbE/2
  Constant4/1 -> one_GbE/8
  one_GbE/1 -> Terminator8/1
  one_GbE/3 -> Terminator1/1
  one_GbE/4 -> Terminator2/1
  one_GbE/5 -> Terminator3/1
  one_GbE/6 -> Terminator4/1
  one_GbE/7 -> Terminator5/1
  one_GbE/8 -> Terminator6/1
  one_GbE/9 -> Terminator7/1
  one_GbE/10 -> Terminator9/1
  one_GbE/11 -> Terminator10/1
  [adc5g] -> Slice2/1
  Constant5/1 -> adc_scope0/2(we), adc_scope0/3(trig)
  Slice2/1 -> adc_scope0/1(din)
  [adc5g] -> Slice3/1
  Constant6/1 -> adc_scope1/3(trig), adc_scope1/2(we)
  Slice3/1 -> adc_scope1/1(din)
  bus_expand2/11(out6) -> Gateway Out23/1
  bus_expand2/12(out5) -> Gateway Out24/1
  bus_expand2/2(out15) -> Gateway Out14/1
  bus_expand2/3(out14) -> Gateway Out15/1
  bus_expand2/5(out12) -> Gateway Out17/1
  bus_expand2/4(out13) -> Gateway Out16/1
  bus_expand2/8(out9) -> Gateway Out20/1
  bus_expand2/15(out2) -> Gateway Out27/1
  bus_expand2/14(out3) -> Gateway Out26/1
  bus_expand2/16(lsb_out1) -> Gateway Out28/1
  bus_expand2/10(out7) -> Gateway Out22/1
  bus_expand2/7(out10) -> Gateway Out19/1
  bus_expand2/9(out8) -> Gateway Out21/1
  bus_expand2/1(msb_out16) -> Gateway Out13/1
  bus_expand2/6(out11) -> Gateway Out18/1
  bus_expand2/13(out4) -> Gateway Out25/1
  [adc_in] -> bus_expand2/1(bus_in)
  bus_expand3/2(out7) -> Gateway Out31/1
  bus_expand3/5(out4) -> Gateway Out34/1
  bus_expand3/3(out6) -> Gateway Out32/1
  bus_expand3/4(out5) -> Gateway Out33/1
  bus_expand3/8(lsb_out1) -> Gateway Out37/1
  bus_expand3/1(msb_out8) -> Gateway Out30/1
  bus_expand3/7(out2) -> Gateway Out36/1
  bus_expand3/6(out3) -> Gateway Out35/1
  Gateway Out29/1 -> ScopeFFT/1
  Gateway Out30/1 -> ScopeFFT/2
  Gateway Out31/1 -> ScopeFFT/3
  Gateway Out32/1 -> ScopeFFT/4
  Gateway Out33/1 -> ScopeFFT/5
  Gateway Out34/1 -> ScopeFFT/6
  Gateway Out35/1 -> ScopeFFT/7
  Gateway Out36/1 -> ScopeFFT/8
  Gateway Out37/1 -> ScopeFFT/9
  Gateway Out1/1 -> ScopeGBE/1
  Gateway Out2/1 -> ScopeGBE/2
  Gateway Out3/1 -> ScopeGBE/3
  fft_shift/1(in_reg) -> Delay21/1
  sim_fftshift/1 -> fft_shift/1(sim_1)
  [reset] -> Gateway Out4/1
  Gateway Out13/1 -> ScopeIn/2
  Gateway Out14/1 -> ScopeIn/3
  Gateway Out15/1 -> ScopeIn/4
  Gateway Out16/1 -> ScopeIn/5
  Gateway Out17/1 -> ScopeIn/6
  Gateway Out18/1 -> ScopeIn/7
  Gateway Out19/1 -> ScopeIn/8
  Gateway Out20/1 -> ScopeIn/9
  Gateway Out21/1 -> ScopeIn/10
  Gateway Out22/1 -> ScopeIn/11
  Gateway Out23/1 -> ScopeIn/12
  Gateway Out24/1 -> ScopeIn/13
  Gateway Out25/1 -> ScopeIn/14
  Gateway Out26/1 -> ScopeIn/15
  Gateway Out27/1 -> ScopeIn/16
  Gateway Out28/1 -> ScopeIn/17
  Gateway Out4/1 -> ScopeIn/1
  Sine Wave2/1 -> asiaa_adc5g/2(sim_c)
  Delay2/1 -> conv_obbus/1(i0)
  Delay3/1 -> conv_obbus/2(i1)
  Delay4/1 -> conv_obbus/3(i2)
  Delay5/1 -> conv_obbus/4(i3)
  Delay6/1 -> conv_obbus/5(i4)
  Delay7/1 -> conv_obbus/6(i5)
  Delay8/1 -> conv_obbus/7(i6)
  Delay9/1 -> conv_obbus/8(i7)
  Delay10/1 -> conv_obbus/9(i8)
  Delay11/1 -> conv_obbus/10(i9)
  Delay12/1 -> conv_obbus/11(i10)
  Delay13/1 -> conv_obbus/12(i11)
  Delay14/1 -> conv_obbus/13(i12)
  Delay15/1 -> conv_obbus/14(i13)
  Delay16/1 -> conv_obbus/15(i14)
  Delay17/1 -> conv_obbus/16(i15)
  Delay18/1 -> [dest_ip]
  Delay19/1 -> edge_detect/1
  Delay20/1 -> [dest_port]
  Delay21/1 -> [fft_shift]
  Delay22/1 -> [bitsel]
  Delay23/1 -> [gain0]
  Delay24/1 -> [gain1]
  Delay25/1 -> [acc_len]
  Delay26/1 -> status/4(out_fft_overflow)
  Delay27/1 -> status/3(out_pkt_overrun)
  Delay28/1 -> status/2(out_tx_overrun)
  Delay29/1 -> [adc5g]
  [reset1] -> one_GbE/1
  Delay30/1 -> [reset1]
  [PPSARMReset] -> TVG/18(sync_in)
  TVG/17(sync_out) -> [reset]
  [adc5g] -> bus_expand4/1(bus_in)
  [use_tvg] -> TVG/1(use_tvg)
  bus_expand4/4(out13) -> TVG/5(adc_i3)
  bus_expand4/5(out12) -> TVG/6(adc_i4)
  bus_expand4/1(msb_out16) -> TVG/2(adc_i0)
  bus_expand4/2(out15) -> TVG/3(adc_i1)
  bus_expand4/3(out14) -> TVG/4(adc_i2)
  bus_expand4/11(out6) -> TVG/12(adc_q2)
  bus_expand4/12(out5) -> TVG/13(adc_q3)
  bus_expand4/15(out2) -> TVG/16(adc_q6)
  bus_expand4/16(lsb_out1) -> TVG/17(adc_q7)
  bus_expand4/6(out11) -> TVG/7(adc_i5)
  bus_expand4/7(out10) -> TVG/8(adc_i6)
  bus_expand4/8(out9) -> TVG/9(adc_i7)
  bus_expand4/9(out8) -> TVG/10(adc_q0)
  bus_expand4/10(out7) -> TVG/11(adc_q1)
  bus_expand4/13(out4) -> TVG/14(adc_q4)
  bus_expand4/14(out3) -> TVG/15(adc_q5)
  TVG/11(q2) -> bus_create3/11(in11)
  TVG/13(q4) -> bus_create3/13(in13)
  TVG/8(i7) -> bus_create3/8(in8)
  TVG/5(i4) -> bus_create3/5(in5)
  TVG/7(i6) -> bus_create3/7(in7)
  TVG/3(i2) -> bus_create3/3(in3)
  TVG/4(i3) -> bus_create3/4(in4)
  TVG/16(q7) -> bus_create3/16(in16)
  TVG/2(i1) -> bus_create3/2(in2)
  TVG/9(q0) -> bus_create3/9(in9)
  TVG/14(q5) -> bus_create3/14(in14)
  TVG/1(i0) -> bus_create3/1(in1)
  TVG/15(q6) -> bus_create3/15(in15)
  TVG/12(q3) -> bus_create3/12(in12)
  TVG/10(q1) -> bus_create3/10(in10)
  TVG/6(i5) -> bus_create3/6(in6)
  bus_create3/1(bus_out) -> [adc_in]
  sim_usetvg/1 -> use_tvg/1(sim_1)
  use_tvg/1(in_reg) -> Delay31/1
  Delay31/1 -> [use_tvg]
  Delay32/1 -> bus_expand1/1(bus_in), scope0/1(din)
  Delay33/1 -> scope1/1(din)
  bus_expand1/1(msb_out4) -> Gateway Out6/1
  bus_expand1/2(out3) -> Gateway Out5/1
  bus_expand1/3(out2) -> Gateway Out7/1
  bus_expand1/4(lsb_out1) -> Gateway Out8/1
  Gateway Out6/1 -> ScopeFFT1/1
  Gateway Out5/1 -> ScopeFFT1/2
  Gateway Out7/1 -> ScopeFFT1/3
  Gateway Out8/1 -> ScopeFFT1/4
  Gateway Out9/1 -> ScopeFFT1/5
  Gateway Out10/1 -> ScopeFFT1/6
  step_arm/1 -> arm/1(sim_1)
  arm/1(in_reg) -> Delay34/1
  ppsarm/1(PPSARMReset) -> [PPSARMReset]
  [sys_reset] -> ppsarm/1(reset)
  [PPS] -> ppsarm/2(PPS)
  [ARM] -> ppsarm/3(ARM)
  Delay34/1 -> [ARM]
  asiaa_adc5g/17(sync_out) -> [PPS]
